simulink model "BMSModel"  (R2024b)
settings: Solver=VariableStepAuto  StartTime=0.0  StopTime=+inf  RelTol=1e-3
values from neighbouring files (symbols this model uses):
  CellCurrentLimitThreshold_Fault = 50    [startInit.m]
  CellCurrentLimitThreshold_Warning = 20    [startInit.m]
  CellTemperatureLimitThreshold_Fault = 65    [startInit.m]
  CellTemperatureLimitThreshold_Warning = 50    [startInit.m]
  CellVoltageLimitHigh_Fault = 4.25    [startInit.m]
  CellVoltageLimitHigh_Warning = 4.21    [startInit.m]
  CellVoltageLimitLow_Fault = 2.65    [startInit.m]
  CellVoltageLimitLow_Warning = 2.70    (= 2.7)    [startInit.m]
  ChargerParRes = 10000    [startInit.m]
  ChargerRes = 0    [startInit.m]
  DisChargerRes = 250    [startInit.m]
  DisInverterRes = 250    [startInit.m]
  DrivetrainEnDelay = 0.1    [startInit.m]
  InverterParRes = 10000    [startInit.m]
  InverterRes = 0    [startInit.m]
  PreChargerRes = 1000    [startInit.m]
  PreInverterRes = 1000    [startInit.m]
  VbattMin = 1    [startInit.m]
  VbattThersholdChrg = 0.8    [startInit.m]
  VbattThresholdDis = 0.2    [startInit.m]
undefined symbols (not defined in the file or in its folder): ContactorCmd, DisChrgCmd, DrivetrainEn, PassiveBalancingRes, PreChrgCmd, sigbuilder_block
libraries: fl_lib x135, nesl_utility x91, deeplib x11, batteryecm_lib x10, ee_lib x10
(part 1 of 13)
settings Model: SimulationMode=accelerator  Creator=E14  LastModifiedBy=E14
settings SolverCC: ZeroCrossAlgorithm=Adaptive
settings DebuggingCC: MaskedZcDiagnostic=none  IgnoredZcDiagnostic=none

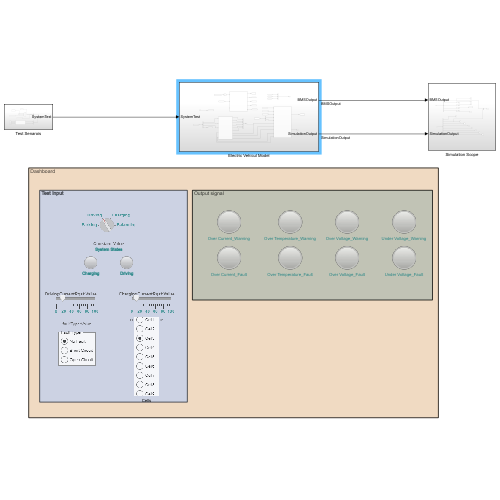
[thumbnail: Simulink's stored preview of the top-level diagram]
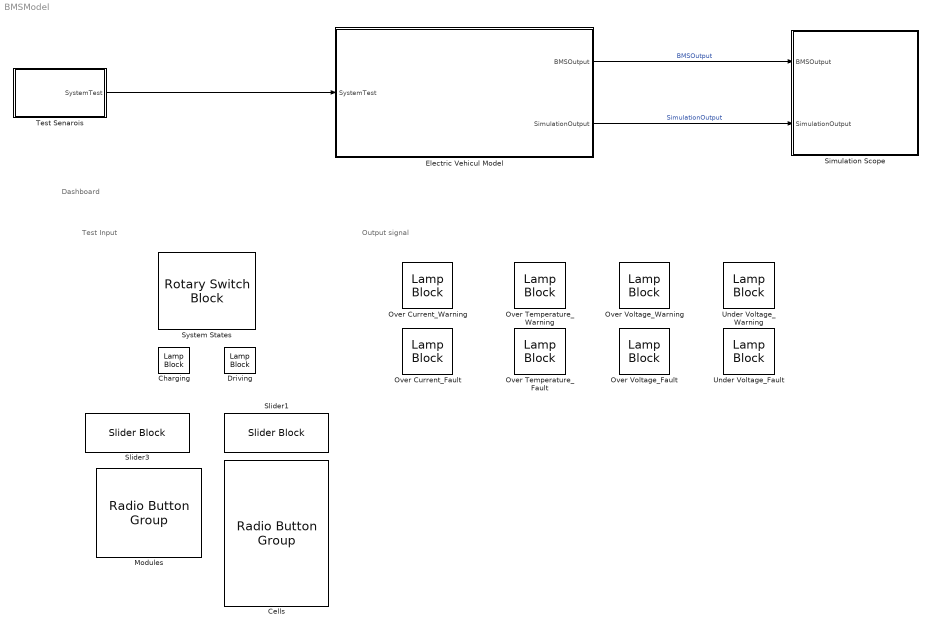
== system BMSModel ==
  note: Output signal
  note: Test Input
  note: Dashboard
  Cells : RadioButtonGroup  SelectedLabel=Cell3  ButtonGroupName=Cells
      settings: Values={{'Cell1', 'Cell2', 'Cell3', 'Cell4', 'Cell5', 'Cell6', 'Cell7', 'Cell8', 'Cell9', 'Cell10'}, [0 1 2 3 4 5 6 7 8 9]}
  Charging : LampBlock  LabelPosition=Hide
  Driving : LampBlock  LabelPosition=Hide
  Electric Vehicul Model : Subsystem
  Modules : RadioButtonGroup  SelectedLabel=No Fault  ButtonGroupName=Fault Type
      settings: Values={{'No Fault', 'Short Circuit', 'Open Circuit'}, [0 1 2]}
  Over Current_Fault : LampBlock  LabelPosition=Hide
  Over Current_Warning : LampBlock  LabelPosition=Hide
  Over Temperature_Fault : LampBlock  LabelPosition=Hide
  Over Temperature_Warning : LampBlock  LabelPosition=Hide
  Over Voltage_Fault : LampBlock  LabelPosition=Hide
  Over Voltage_Warning : LampBlock  LabelPosition=Hide
  Simulation Scope : Subsystem
  Slider1 : SliderBlock
  Slider3 : SliderBlock
  System States : RotarySwitchBlock  LabelPosition=Bottom
      settings: Values={{'Parking', 'Driving', 'Charging', 'Balancing'}, [0 1 2 3]}
  Test Senarois : Subsystem
  Under Voltage_Fault : LampBlock  LabelPosition=Hide
  Under Voltage_Warning : LampBlock  LabelPosition=Hide
  wires:
  Electric Vehicul Model/1(BMSOutput) -> Simulation Scope/1(BMSOutput)  "BMSOutput"
  Electric Vehicul Model/2(SimulationOutput) -> Simulation Scope/2(SimulationOutput)  "SimulationOutput"
  Test Senarois/1(SystemTest) -> Electric Vehicul Model/1(SystemTest)

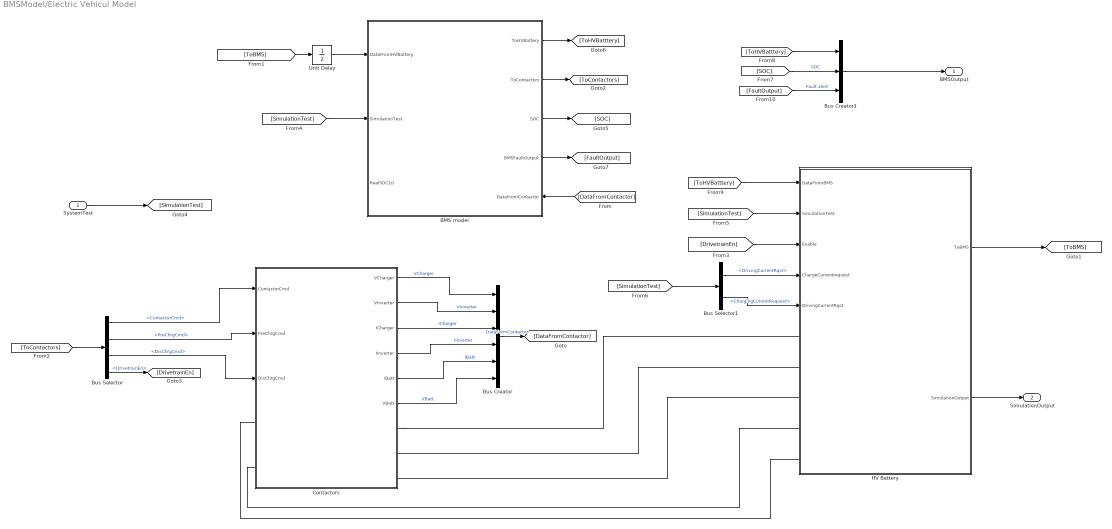
== system BMSModel/Electric Vehicul Model ==
  SystemTest : Inport
  BMS model : Subsystem
      settings: PortSchema="{\"entries\":[{\"content\":{\"sides\":[{\"content\":{\"connectorIds\":[\"In1\",\"In2\",\"In3\"]},\"type\":\"ConnectorPlacement.EquallySpacedRectSide\",\"uuid\":\"2c589a25-f261-4fce-a2ec-292fd7546e04\"},{\"content\":{\"connectorIds\":[\"Out1\",\"Out2\",\"Out3\",\"Out4\",\"In4\"],\"side\":\"RIGHT\"},\"type\":\"ConnectorPlacement.EquallySpacedRectSide\",\"uuid\":\"636110e9-c19f-45ef-a995-7063c21b24c5\"},{\"content\":{\"side\":\"TOP\"},\"type\":\"ConnectorPlacement.EquallySpacedRectSide\",\"uuid\":\"0f79ccdb-6976-4435-9408-571257b8b1d1\"}]},\"type\":\"ConnectorPlacement.EquallySpacedConnector\",\"uuid\":\"4eb207e4-2090-47bd-85fa-5a88c7712e39\"}],\"packageUris\":[\"http://schema.mathworks.com/mf0/Connector/R2020b\"],\"version\":\"1.0\"}"
  Bus Creator : BusCreator  Inputs=6
  Bus Creator1 : BusCreator  Inputs=3
  Bus Selector : BusSelector  OutputSignals=ContactorCmd,PreChrgCmd,DisChrgCmd,DrivetrainEn
  Bus Selector1 : BusSelector  OutputSignals=SimulationSystemInput.DrivingCurrentRqst,SimulationSystemInput.ChargingCurrentRequest
  Contactors : Subsystem
  HV Battery : Subsystem
  Unit Delay : UnitDelay  SampleTime=-1  HasFrameUpgradeWarning=on
  BMSOutput : Outport
  SimulationOutput : Outport  Port=2
  wires:
  SystemTest/1 -> [SimulationTest]
  HV Battery/2(SimulationOutput) -> SimulationOutput/1
  Contactors/1(VCharger) -> Bus Creator/1  "VCharger"
  Contactors/2(VInverter) -> Bus Creator/2  "VInverter"
  Contactors/3(ICharger) -> Bus Creator/3  "ICharger"
  Contactors/4(IInverter) -> Bus Creator/4  "IInverter"
  Contactors/5(IBatt) -> Bus Creator/5  "IBatt"
  Contactors/6(VBatt) -> Bus Creator/6  "VBatt"
  BMS model/2(ToContactors) -> [ToContactors]
  Bus Selector/1 -> Contactors/1(ContactorCmd)  "<ContactorCmd>"
  Bus Selector/2 -> Contactors/2(PreChrgCmd)  "<PreChrgCmd>"
  Bus Selector/3 -> Contactors/3(DisChrgCmd)  "<DisChrgCmd>"
  Bus Selector1/1 -> HV Battery/4(ChargeCurrentrequest)  "<DrivingCurrentRqst>"
  Bus Selector1/2 -> HV Battery/5(DrivingCurrentRqst)  "<ChargingCurrentRequest>"
  Bus Creator1/1 -> BMSOutput/1
  [DataFromContactor] -> BMS model/4(DataFromContactor)
  Bus Creator/1 -> [DataFromContactor]  "DataFormContactor"
  HV Battery/1(ToBMS) -> [ToBMS]
  [ToContactors] -> Bus Selector/1
  Bus Selector/4 -> [DrivetrainEn]  "<DrivetrainEn>"
  [DrivetrainEn] -> HV Battery/3(Enable)
  [SimulationTest] -> BMS model/2(SimulationTest)
  [SimulationTest] -> Bus Selector1/1
  [SimulationTest] -> HV Battery/2(SimulationTest)
  BMS model/3(SOC) -> [SOC]
  [SOC] -> Bus Creator1/2  "SOC"
  BMS model/1(ToHVBattery) -> [ToHVBatttery]
  [ToHVBatttery] -> Bus Creator1/1
  [ToHVBatttery] -> HV Battery/1(DataFromBMS)
  BMS model/4(BMSFaultOutput) -> [FaultOutput]
  [FaultOutput] -> Bus Creator1/3  "Fault alert"
  [ToBMS] -> Unit Delay/1
  Unit Delay/1 -> BMS model/1(DataFromHVBattery)
  connections:
  Contactors/RConn1 -- HV Battery/LConn1
  HV Battery/LConn5 -- Contactors/LConn1
  HV Battery/LConn2 -- Contactors/RConn2
  HV Battery/LConn3 -- Contactors/RConn3
  HV Battery/LConn4 -- Contactors/LConn2

== system BMSModel/Simulation Scope ==
  BMSOutput : Inport
  SimulationOutput : Inport  Port=2
  Balancing DeltaV Cell : Scope
  Balancing Flags : Display
  Ballancing : Scope
  Bus Selector : BusSelector  OutputSignals=SOC,Balancing.balancingDeltaVCell,Balancing.balancingCmd,Balancing.balancingFlags,Fault alert
  Bus Selector1 : BusSelector  OutputSignals=BusInputBMS.ModelTempVector,BusInputBMS.ModelRealSOCVector,BusInputBMS.ModelVoltageVector,BusInputBMS.ModelCurrentVector,signal2.ChargerCurrentSensor,signal2.ChargingVoltageSensor,signal2.InverterCurrentSensor,signal2.InverterVoltageSensor
  Charge Currrent : Scope
  Charge Voltage : Scope
  Fault alert : Scope
  Inv Current : Scope
  Inv Voltage : Scope
  Mux : Mux  Inputs=2
  Real Currrent : Scope
  Real SOC : Scope
  Real Voltage : Scope
  SOC_Estimate : Scope
  SOC_Estimate1 : Scope
  TempVector : Scope
  Terminator{1,10..12} (4 alike) : Terminator
  wires:
  SimulationOutput/1 -> Bus Selector1/1
  BMSOutput/1 -> Bus Selector/1
  Bus Selector1/8 -> Inv Voltage/1  "<InverterVoltageSensor>"
  Bus Selector1/7 -> Inv Current/1  "<InverterCurrentSensor>"
  Bus Selector1/6 -> Charge Voltage/1  "<ChargingVoltageSensor>"
  Bus Selector1/5 -> Charge Currrent/1  "<ChargerCurrentSensor>"
  Bus Selector1/4 -> Real Currrent/1  "<ModelCurrentVector>"
  Bus Selector1/3 -> Real Voltage/1  "<ModelVoltageVector>"
  Bus Selector1/2 -> Mux/2, Real SOC/1  "<ModelRealSOCVector>"
  Bus Selector1/1 -> TempVector/1  "<ModelTempVector>"
  Bus Selector/1 -> Mux/1, SOC_Estimate/1  "<SOC>"
  Bus Selector/2 -> Balancing DeltaV Cell/1, Terminator1/1  "<balancingDeltaVCell>"
  Bus Selector/3 -> Ballancing/1, Terminator10/1  "<balancingCmd>"
  Bus Selector/4 -> Balancing Flags/1, Terminator11/1  "<balancingFlags>"
  Bus Selector/5 -> Fault alert/1, Terminator12/1  "<Fault alert>"
  Mux/1 -> SOC_Estimate1/1

== system BMSModel/Test Senarois ==
  Bus Creator : BusCreator  Inputs=3
  Bus Creator1 : BusCreator  Inputs=2
  Bus Creator2 : BusCreator  Inputs=2
  ChargingCurrentRqst : Constant  Value=11.02756892230576
  Constant : Constant
  DrivingCurrentRqst : Constant  Value=17.04260651629072
  DrivingCurrentSelector : Constant
  MATLAB Function : MATLAB Function  TreatAsAtomicUnit=on
  ModelNumber : Constant
  Signal Builder : Subsystem  Tag=STV Subsys  LoadFcn=sigbuilder_block('load');  StartFcn=sigbuilder_block('start');  StopFcn=sigbuilder_block('stop');  ClipboardFcn=sigbuilder_block('clipboard');  mask(Sigbuilder block){tuvar=[0:10]}
      mask help: Simulink:masks:SigbuilderBlock_MD
      mask init:
      | if ~strcmp(get_param(bdroot(gcbh),'SimulationStatus'),'stopped') tuvar = sigbuilder_block('maskInit'); end
      settings: CopyFcn=sigbuilder_block('copy');  DeleteFcn=sigbuilder_block('delete');  ModelCloseFcn=sigbuilder_block('modelClose');  PreSaveFcn=sigbuilder_block('preSave');  PauseFcn=sigbuilder_block('pause');  ContinueFcn=sigbuilder_block('continue');  NameChangeFcn=sigbuilder_block('namechange');  OpenFcn=sigbuilder_block('open',[5.4 5.4 1141.2 580.8 ]);  CloseFcn=sigbuilder_block('close');
      mask Display: plot(0, 0, 100, 100,[2, 2, 32, 32, 2], [68, 8, 8, 68, 68],[32, 2], [38, 38], [32, 19, 2],[53, 60, 44], [32, 17, 17, 2],[16, 16, 31, 31]);txt = getActiveGroup(gcbh);text(2, 100, txt,'verticalAlignment', 'top');
  Switch : Switch  Threshold=2  SaturateOnIntegerOverflow=off  InputSameDT=off
  cellNumber : Constant  Value=2
  faultType : Constant  Value=0
  SystemTest : Outport
  wires:
  faultType/1 -> MATLAB Function/1(faultType)
  ChargingCurrentRqst/1 -> Bus Creator/3  "ChargingCurrentRequest"
  Constant/1 -> Bus Creator/1  "SystemState"
  Bus Creator1/1 -> Bus Creator2/2  "SimulationBatteryPackInput"
  DrivingCurrentSelector/1 -> Switch/2
  Signal Builder/1(Current) -> Switch/1
  MATLAB Function/1(SCInjVector) -> Bus Creator1/1  "SCInjection"
  DrivingCurrentRqst/1 -> Switch/3
  Switch/1 -> Bus Creator/2  "DrivingCurrentRqst"
  MATLAB Function/2(OCInjVector) -> Bus Creator1/2  "OCInjection"
  Bus Creator/1 -> Bus Creator2/1  "SimulationSystemInput"
  cellNumber/1 -> MATLAB Function/3(cellNumber)
  ModelNumber/1 -> MATLAB Function/2(ModelNumber)
  Bus Creator2/1 -> SystemTest/1  "BusSimulationInput"

== system BMSModel/Electric Vehicul Model/BMS model ==
  DataFromHVBattery : Inport
  SimulationTest : Inport  Port=2
  RealSOC10 : Inport  Port=3
  DataFromContactor : Inport  Port=4
  Balancing system : Subsystem
  Bus Creator : BusCreator  Inputs=3
  Bus Selector : BusSelector  OutputSignals=ModelVoltageVector
  Bus Selector1 : BusSelector  OutputSignals=SimulationSystemInput.SystemState
  Bus Selector2 : BusSelector  OutputSignals=ModelCurrentVector,ModelTempVector,ModelVoltageVector
  Bus Selector3 : BusSelector  OutputSignals=ModelCurrentVector,ModelTempVector,ModelVoltageVector
  Contactor commands : Subsystem
  Faults detection : Subsystem
  SOC_Estimation : Subsystem
      settings: PortSchema="{\"entries\":[{\"content\":{\"sides\":[{\"content\":{\"connectorIds\":[\"In1\",\"In2\",\"In3\"]},\"type\":\"ConnectorPlacement.EquallySpacedRectSide\",\"uuid\":\"876fcd95-3065-43cb-b3a9-db79c29902f5\"},{\"content\":{\"connectorIds\":[\"Out1\"],\"side\":\"RIGHT\"},\"type\":\"ConnectorPlacement.EquallySpacedRectSide\",\"uuid\":\"b84fbb32-82b4-42f9-ac0b-0590da1d8a8e\"},{\"content\":{\"side\":\"TOP\"},\"type\":\"ConnectorPlacement.EquallySpacedRectSide\",\"uuid\":\"c1f6d7e2-5dfd-4d06-ab5c-e56c33ab6eb0\"},{\"content\":{\"connectorIds\":[\"In4\"],\"side\":\"BOTTOM\"},\"type\":\"ConnectorPlacement.EquallySpacedRectSide\",\"uuid\":\"ce994544-dd90-4c11-8c2e-7269739abe7d\"}]},\"type\":\"ConnectorPlacement.EquallySpacedConnector\",\"uuid\":\"5e5b4979-c97b-4b47-85d5-3773cafc806b\"}],\"packageUris\":[\"http://schema.mathworks.com/mf0/Connector/R2020b\"],\"version\":\"1.0\"}"
  ToHVBattery : Outport
  ToContactors : Outport  Port=2
  SOC : Outport  Port=3
  BMSFaultOutput : Outport  Port=4
  wires:
  DataFromHVBattery/1 -> Bus Selector/1, [ToFaul], Bus Selector2/1
  Bus Selector/1 -> Balancing system/2(VoltageVector)  "<ModelVoltageVector>"
  SimulationTest/1 -> Bus Selector1/1
  Bus Selector1/1 -> Balancing system/1(SystemState), Contactor commands/1(SystemState)  "<SystemState>"
  Balancing system/1(balancingCmd) -> Bus Creator/1  "balancingCmd"
  Balancing system/2(balancingDeltaVCell) -> Bus Creator/2  "balancingDeltaVCell"
  Balancing system/3(balancingFlags) -> Bus Creator/3  "balancingFlags"
  Bus Creator/1 -> ToHVBattery/1  "Balancing"
  Bus Selector2/1 -> SOC_Estimation/1(CurrentVector)  "<ModelCurrentVector>"
  Bus Selector2/3 -> SOC_Estimation/3(VoltageVector)  "<ModelVoltageVector>"
  Bus Selector2/2 -> SOC_Estimation/2(TempVector)  "<ModelTempVector>"
  Contactor commands/1(ContactorModuleCmd) -> ToContactors/1
  SOC_Estimation/1(Out1) -> SOC/1
  DataFromContactor/1 -> Contactor commands/2(ContactorModuleData)
  Faults detection/1(BMSFaultOutput) -> BMSFaultOutput/1
  [ToFaul] -> Bus Selector3/1
  Bus Selector3/2 -> Faults detection/2(<TempVector>)  "<ModelTempVector>"
  Bus Selector3/1 -> Faults detection/1(<CurrentVector>)  "<ModelCurrentVector>"
  Bus Selector3/3 -> Faults detection/3(<VoltageVector>)  "<ModelVoltageVector>"
  RealSOC10/1 -> SOC_Estimation/4(RealSOC10)

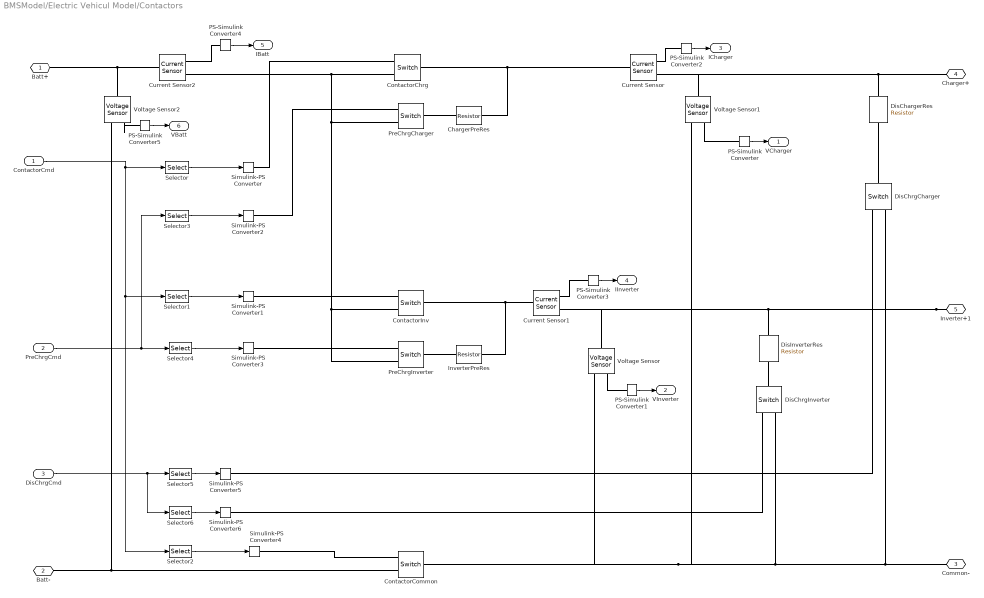
== system BMSModel/Electric Vehicul Model/Contactors ==
  ContactorCmd : Inport
  PreChrgCmd : Inport  Port=2
  DisChrgCmd : Inport  Port=3
  ChargerPreRes : fl_lib/Electrical/Electrical Elements/Resistor  R=PreChargerRes Ohm  i_specify=off  i_priority=None  i=0 A  i_nominal_specify=off  i_nominal_value=1  i_nominal_unit=A  v_specify=off  v_priority=None  v=0 V  v_nominal_specify=off  v_nominal_value=1  v_nominal_unit=V
  ContactorChrg : fl_lib/Electrical/Electrical Elements/Switch  R_closed=ContactorChargerRes Ohm  G_open=1e-8 1/Ohm  Threshold=0.5  i_specify=off  i_priority=None  i=0 A  i_nominal_specify=off  i_nominal_value=1  i_nominal_unit=A  v_specify=off  v_priority=None  v=0 V  v_nominal_specify=off  v_nominal_value=1  v_nominal_unit=V
  ContactorCommon : fl_lib/Electrical/Electrical Elements/Switch  R_closed=ContactorCommonRes Ohm  G_open=1e-8 1/Ohm  Threshold=0.5  i_specify=off  i_priority=None  i=0 A  i_nominal_specify=off  i_nominal_value=1  i_nominal_unit=A  v_specify=off  v_priority=None  v=0 V  v_nominal_specify=off  v_nominal_value=1  v_nominal_unit=V
  ContactorInv : fl_lib/Electrical/Electrical Elements/Switch  R_closed=ContactorInverterRes Ohm  G_open=1e-8 1/Ohm  Threshold=0.5  i_specify=off  i_priority=None  i=0 A  i_nominal_specify=off  i_nominal_value=1  i_nominal_unit=A  v_specify=off  v_priority=None  v=0 V  v_nominal_specify=off  v_nominal_value=1  v_nominal_unit=V
  Current Sensor : fl_lib/Electrical/Electrical Sensors/Current Sensor
  Current Sensor1 : fl_lib/Electrical/Electrical Sensors/Current Sensor
  Current Sensor2 : fl_lib/Electrical/Electrical Sensors/Current Sensor
  DisChargerRes : fl_lib/Electrical/Electrical Elements/Resistor  R=DisChargerRes Ohm  i_specify=off  i_priority=None  i=0 A  i_nominal_specify=off  i_nominal_value=1  i_nominal_unit=A  v_specify=off  v_priority=None  v=0 V  v_nominal_specify=off  v_nominal_value=1  v_nominal_unit=V
  DisChrgCharger : fl_lib/Electrical/Electrical Elements/Switch  R_closed=RelayDisChargerRes Ohm  G_open=1e-8 1/Ohm  Threshold=0.5  i_specify=off  i_priority=None  i=0 A  i_nominal_specify=off  i_nominal_value=1  i_nominal_unit=A  v_specify=off  v_priority=None  v=0 V  v_nominal_specify=off  v_nominal_value=1  v_nominal_unit=V
  DisChrgInverter : fl_lib/Electrical/Electrical Elements/Switch  R_closed=RelayDisInverterRes Ohm  G_open=1e-8 1/Ohm  Threshold=0.5  i_specify=off  i_priority=None  i=0 A  i_nominal_specify=off  i_nominal_value=1  i_nominal_unit=A  v_specify=off  v_priority=None  v=0 V  v_nominal_specify=off  v_nominal_value=1  v_nominal_unit=V
  DisInverterRes : fl_lib/Electrical/Electrical Elements/Resistor  R=DisInverterRes Ohm  i_specify=off  i_priority=None  i=0 A  i_nominal_specify=off  i_nominal_value=1  i_nominal_unit=A  v_specify=off  v_priority=None  v=0 V  v_nominal_specify=off  v_nominal_value=1  v_nominal_unit=V
  InverterPreRes : fl_lib/Electrical/Electrical Elements/Resistor  R=PreInverterRes Ohm  i_specify=off  i_priority=None  i=0 A  i_nominal_specify=off  i_nominal_value=1  i_nominal_unit=A  v_specify=off  v_priority=None  v=0 V  v_nominal_specify=off  v_nominal_value=1  v_nominal_unit=V
  PS-Simulink Converter{,1..5} (6 alike) : nesl_utility/PS-Simulink Converter  PhysicalDomain=network_engine_domain  SubClassName=ps_output  LeftPortType=input  RightPortType=output  PseudoPeriodic=off  Frequencies=[]  VectorFormat=1-D array  Unit=inherit  AffineConversion=off
  PreChrgCharger : fl_lib/Electrical/Electrical Elements/Switch  R_closed=RelayPreChargerRes Ohm  G_open=1e-8 1/Ohm  Threshold=0.5  i_specify=off  i_priority=None  i=0 A  i_nominal_specify=off  i_nominal_value=1  i_nominal_unit=A  v_specify=off  v_priority=None  v=0 V  v_nominal_specify=off  v_nominal_value=1  v_nominal_unit=V
  PreChrgInverter : fl_lib/Electrical/Electrical Elements/Switch  R_closed=RelayPreInverterRes Ohm  G_open=1e-8 1/Ohm  Threshold=0.5  i_specify=off  i_priority=None  i=0 A  i_nominal_specify=off  i_nominal_value=1  i_nominal_unit=A  v_specify=off  v_priority=None  v=0 V  v_nominal_specify=off  v_nominal_value=1  v_nominal_unit=V
  Selector : Selector  InputPortWidth=3  IndexOptions=Index vector (dialog)  Indices=1  OutputSizes=1
  Selector1 : Selector  InputPortWidth=3  IndexOptions=Index vector (dialog)  Indices=2  OutputSizes=1
  Selector2 : Selector  InputPortWidth=3  IndexOptions=Index vector (dialog)  Indices=3  OutputSizes=1
  Selector3 : Selector  InputPortWidth=2  IndexOptions=Index vector (dialog)  Indices=1  OutputSizes=1
  Selector4 : Selector  InputPortWidth=2  IndexOptions=Index vector (dialog)  Indices=2  OutputSizes=1
  Selector5 : Selector  InputPortWidth=2  IndexOptions=Index vector (dialog)  Indices=1  OutputSizes=1
  Selector6 : Selector  InputPortWidth=2  IndexOptions=Index vector (dialog)  Indices=2  OutputSizes=1
  Simulink-PS Converter{,1..6} (7 alike) : nesl_utility/Simulink-PS Converter  PhysicalDomain=network_engine_domain  SubClassName=ps_input  LeftPortType=input  RightPortType=output  PseudoPeriodic=off  Frequencies=[]  NoiseDistribution=none  NoiseParameters=[]  Unit=1  AffineConversion=off  FilteringAndDerivatives=provide  SimscapeFilterOrder=1  InputFilterTimeConstant=0.001  UdotUserProvided=0
  Voltage Sensor : fl_lib/Electrical/Electrical Sensors/Voltage Sensor
  Voltage Sensor1 : fl_lib/Electrical/Electrical Sensors/Voltage Sensor
  Voltage Sensor2 : fl_lib/Electrical/Electrical Sensors/Voltage Sensor
  Batt+ : PMIOPort  Side=Left
  Batt- : PMIOPort  Port=2  Side=Left
  Common- : PMIOPort  Port=3  Side=Right
  Charger+ : PMIOPort  Port=4  Side=Right
  Inverter+1 : PMIOPort  Port=5  Side=Right
  VCharger : Outport
  VInverter : Outport  Port=2
  ICharger : Outport  Port=3
  IInverter : Outport  Port=4
  IBatt : Outport  Port=5
  VBatt : Outport  Port=6
  wires:
  PS-Simulink Converter/1 -> VCharger/1
  ContactorCmd/1 -> Selector2/1, Selector1/1, Selector/1
  PS-Simulink Converter5/1 -> VBatt/1
  Selector4/1 -> Simulink-PS Converter3/1
  Selector2/1 -> Simulink-PS Converter4/1
  PS-Simulink Converter4/1 -> IBatt/1
  Selector6/1 -> Simulink-PS Converter6/1
  PS-Simulink Converter1/1 -> VInverter/1
  Selector/1 -> Simulink-PS Converter/1
  Selector5/1 -> Simulink-PS Converter5/1
  PreChrgCmd/1 -> Selector3/1, Selector4/1
  Selector1/1 -> Simulink-PS Converter1/1
  DisChrgCmd/1 -> Selector6/1, Selector5/1
  PS-Simulink Converter2/1 -> ICharger/1
  PS-Simulink Converter3/1 -> IInverter/1
  Selector3/1 -> Simulink-PS Converter2/1
  connections:
  Voltage Sensor2/LConn1 -- Current Sensor2/LConn1 -- Batt+/RConn1
  Voltage Sensor2/RConn2 -- Batt-/RConn1 -- ContactorCommon/RConn2
  ContactorChrg/LConn1 -- ChargerPreRes/RConn1 -- Current Sensor/LConn1
  Current Sensor2/RConn2 -- ContactorChrg/RConn2 -- PreChrgCharger/RConn2 -- ContactorInv/RConn2 -- PreChrgInverter/RConn2
  DisChrgCharger/RConn2 -- Common-/RConn1 -- DisChrgInverter/RConn2 -- Voltage Sensor1/RConn2 -- ContactorCommon/LConn1 -- Voltage Sensor/RConn2
  Inverter+1/RConn1 -- DisInverterRes/LConn1 -- Current Sensor1/RConn2 -- Voltage Sensor/LConn1
  InverterPreRes/RConn1 -- Current Sensor1/LConn1 -- ContactorInv/LConn1
  DisChargerRes/LConn1 -- Charger+/RConn1 -- Current Sensor/RConn2 -- Voltage Sensor1/LConn1
  Voltage Sensor/RConn1 -- PS-Simulink Converter1/LConn1
  DisChrgCharger/LConn1 -- DisChargerRes/RConn1
  DisChrgCharger/RConn1 -- Simulink-PS Converter5/RConn1
  PreChrgCharger/LConn1 -- ChargerPreRes/LConn1
  Simulink-PS Converter1/RConn1 -- ContactorInv/RConn1
  Current Sensor2/RConn1 -- PS-Simulink Converter4/LConn1
  Simulink-PS Converter3/RConn1 -- PreChrgInverter/RConn1
  PreChrgInverter/LConn1 -- InverterPreRes/LConn1
  Simulink-PS Converter2/RConn1 -- PreChrgCharger/RConn1
  DisChrgInverter/LConn1 -- DisInverterRes/RConn1
  Simulink-PS Converter/RConn1 -- ContactorChrg/RConn1
  Simulink-PS Converter4/RConn1 -- ContactorCommon/RConn1
  Voltage Sensor2/RConn1 -- PS-Simulink Converter5/LConn1
  Simulink-PS Converter6/RConn1 -- DisChrgInverter/RConn1
  Current Sensor1/RConn1 -- PS-Simulink Converter3/LConn1
  Voltage Sensor1/RConn1 -- PS-Simulink Converter/LConn1
  Current Sensor/RConn1 -- PS-Simulink Converter2/LConn1
